simulink library "Renault_Library"  (Simulink 8.2)
settings: Solver=ode3  StartTime=0.0  StopTime=10.0  FixedStep=0.020  RelTol=1e-3
undefined symbols (not defined in the file or in its folder): ABC, AssystemProposal, b, chkval, CoeffA_C, CoeffB_C, CoeffMean_C, ConfTime_C, DiagDelay_C, DividePercent, FailureConfActive_C, FallingEdgeDuration_C, HighLevelThresholdVoltage_C, HighLevelVoltage_C, HighLevelVoltageTunable_C, InitValue_C, InputVoltRangeMax_C, InputVoltRangeMin_C, LowActiveContact_C, LowLevelThresholdVoltage_C, LowLevelVoltage_C, MaxOperatingVoltage_C, MinOperatingVoltage_C, mV2V_conversion, OUT1, OutOfRangeHigh_C, OutOfRangeLow_C, PinSupplySaturationMax, PinSupplySaturationMin, Res1Value_C, Res2Value_C, Res3Value_C, RisingEdgeDuration_C, RSA_CANSignalFailMonitoring, SampleTime_C, service14Table, service19Table, service22Table, service24Table, service28Table, service2ETable, service2FTable, service31Table, service87Table, SizeXStateDID2FTable, SizeXTable14, SizeXTable19, SizeXTable22, SizeXTable24, SizeXTable28, SizeXTable2E, SizeXTable2F, SizeXTable31, SizeXTable87, SizeYStateDID2FTable, SizeYTable14, SizeYTable19, SizeYTable22, SizeYTable24, SizeYTable28 ... (+22)
undefined symbols (not defined in the file): BatteryVoltage_ActivationThreshold_C, FrameStatus_AbsentValue_C, FrameStatus_ConfAbsentValue_C, MemThreshold_C, NAMEOFTHEMODEL_SAMPLE_TIME_CST, NbAbs1_C, NbConfAbs1_C, NbConfPres1_C, NbPres1_C, NOMDUSIGNAL_FaultConfTime_C, NOMDUSIGNAL_FaultRehabTime_C, NOMDUSIGNAL_switchvalue_C, NOMDUSIGNAL_unavailablevalue_C, PeriodFrame1_C, ResetTime_C, WakeUpTime_C
libraries: Renault_Library x112, simulink x75
(part 12 of 32)

== system Renault_Library/Complex blocks/Timers & Counters/RSA_TurnOnDelay2_v01_05 ==
  in : Inport
      settings: AttributesFormatString=Type : %<OutDataTypeStr>
  dly : Inport  Port=2
      settings: AttributesFormatString=Type : %<OutDataTypeStr>
  Constant1 : Constant  Value=Ts  OutDataTypeStr=Inherit: Inherit via back propagation  SampleTime=-1
  Constant4 : Constant  Tag=AM,Constant,0,1,Value;CMP,Value;  Value=1000  OutDataTypeStr=uint16  SampleTime=-1
  Constant5 : Constant  Value=INIVAL  OutDataTypeStr=Inherit: Inherit via back propagation  SampleTime=-1
  Constant6 : Constant  Tag=AM,Constant,0,1,Value;CMP,Value;  OutDataTypeStr=Inherit: Inherit via back propagation  SampleTime=-1
  DataTypeConversion1 : DataTypeConversion  OutDataTypeStr=uint32  SaturateOnIntegerOverflow=on  RndMeth=Zero
  DataTypeConversion2 : DataTypeConversion  RndMeth=Zero
  DataTypeConversion3 : DataTypeConversion  RndMeth=Zero
  Logical_Operator : Logic  Operator=NOT  OutDataTypeStr=boolean
  Logical_Operator1 : Logic  OutDataTypeStr=boolean
  Logical_Operator2 : Logic  OutDataTypeStr=boolean
  Logical_Operator6 : Logic  Operator=NOT  OutDataTypeStr=boolean
  Product : Product  SaturateOnIntegerOverflow=on  RndMeth=Zero
  Product1 : Product  SaturateOnIntegerOverflow=on  RndMeth=Zero
  Product2 : Product  Inputs=*/  SaturateOnIntegerOverflow=on  RndMeth=Zero
  RSA_CompareToZero : Renault_Library/Complex blocks/Logic & Bit Operations/RSA_CompareToZero  Tag=[LIB]  relop=~=  LogicOutDataTypeMode=boolean
      settings: AttributesFormatString=%<MaskType> : Ver : 01.03\nOperator: %<relop>\nOutput data type mode: %<LogicOutDataTypeMode>\nEnable zero-crossing detection %<ZeroCross>
  RSA_DataTypeDuplicate_v01_03 : Renault_Library/Complex blocks/Signal Routing/RSA_DataTypeDuplicate_v01_03  Tag=[LIB]  NoIn=2
      settings: AttributesFormatString=%<MaskType> : Ver : 01.03\nNo Of Inputs %<NoIn>
  Relational_Operator1 : RelationalOperator  Operator=<=  OutDataTypeStr=boolean  InputSameDT=on
  Relational_Operator8 : RelationalOperator  Operator=>  OutDataTypeStr=boolean  InputSameDT=on
  Subtract4 : Sum  Inputs=-+  OutDataTypeStr=Inherit: Inherit via back propagation  RndMeth=Zero  SaturateOnIntegerOverflow=on  InputSameDT=on
      settings: AttributesFormatString=SAT = %<SaturateOnIntegerOverflow>
  Sum1 : Sum  Inputs=+-  OutDataTypeStr=Inherit: Inherit via back propagation  RndMeth=Zero  SaturateOnIntegerOverflow=on  InputSameDT=on
      settings: AttributesFormatString=SAT = %<SaturateOnIntegerOverflow>
  Switch1 : Switch  Criteria=u2 ~= 0  RndMeth=Zero  SaturateOnIntegerOverflow=on  InputSameDT=on
      settings: AttributesFormatString=Criteria=%<Criteria>
  Switch2 : Switch  Criteria=u2 ~= 0  RndMeth=Zero  SaturateOnIntegerOverflow=on  InputSameDT=on
      settings: AttributesFormatString=Criteria=%<Criteria>
  Switch3 : Switch  Criteria=u2 ~= 0  RndMeth=Zero  SaturateOnIntegerOverflow=on  InputSameDT=on
      settings: AttributesFormatString=Criteria=%<Criteria>
  Switch5 : Switch  Tag=GEN,Threshold;AM,Switch,2,1;CMP,Threshold;  Criteria=u2 ~= 0  RndMeth=Zero  SaturateOnIntegerOverflow=on  InputSameDT=on
      settings: AttributesFormatString=Criteria=%<Criteria>
  UnitDelay : UnitDelay  InitialCondition=false  SampleTime=-1
      settings: AttributesFormatString=IC = %<X0>
  Unit_Delay2 : UnitDelay  InitialCondition=INIVAL  SampleTime=-1
      settings: AttributesFormatString=IC = %<X0>
  out : Outport
      settings: AttributesFormatString=Type : %<OutDataTypeStr>\nInit :%<InitialOutput>
  ctr : Outport  Port=2
      settings: AttributesFormatString=Type : %<OutDataTypeStr>\nInit :%<InitialOutput>
  wires:
  Constant5/1 -> [INIVAL], Switch1/1
  in/1 -> Logical_Operator1/1, UnitDelay/1, Logical_Operator2/1, Logical_Operator/1
  [dly] -> Switch2/1
  Logical_Operator2/1 -> Switch2/2
  Logical_Operator6/1 -> Logical_Operator2/2
  UnitDelay/1 -> Logical_Operator6/1
  Switch1/1 -> Product2/1, Unit_Delay2/1, Relational_Operator8/2
  Switch2/1 -> Switch1/3
  Logical_Operator1/1 -> out/1
  Relational_Operator8/1 -> Logical_Operator1/2
  Logical_Operator/1 -> Switch1/2
  Unit_Delay2/1 -> Sum1/1, Relational_Operator1/1
  Relational_Operator1/1 -> Switch3/2
  Sum1/1 -> Switch3/3
  [Timestep] -> Sum1/2
  Switch3/1 -> Switch2/3
  [INIVAL] -> Switch3/1
  [Ts] -> DataTypeConversion1/1, Subtract4/2
  DataTypeConversion1/1 -> DataTypeConversion2/1
  DataTypeConversion2/1 -> Subtract4/1
  RSA_CompareToZero/1 -> Switch5/2
  Subtract4/1 -> RSA_CompareToZero/1
  Constant6/1 -> Switch5/3
  Constant4/1 -> Switch5/1
  Switch5/1 -> [tolcons]
  Product/1 -> [Timestep]
  Constant1/1 -> RSA_DataTypeDuplicate_v01_03/2, [Ts], Product/1
  [tolcons] -> Product/2
  [Timestep] -> Relational_Operator1/2
  [Timestep] -> Relational_Operator8/1
  Product1/1 -> [dly]
  [tolcons] -> Product1/1
  dly/1 -> RSA_DataTypeDuplicate_v01_03/1, Product1/2
  DataTypeConversion3/1 -> ctr/1
  Product2/1 -> DataTypeConversion3/1
  [tolcons] -> Product2/2

== system Renault_Library/Complex blocks/Timers & Counters/RSA_TurnOnDelay2_v01_06 ==
  delay_flag : Inport
      settings: AttributesFormatString=Type : %<OutDataTypeStr>
  dly : Inport  Port=2
      settings: AttributesFormatString=Type : %<OutDataTypeStr>
  Constant1 : Constant  Value=Ts  OutDataTypeStr=SampleDT
  Constant2 : Constant  Value=Boosting_Factor  OutDataTypeStr=Constant_DT
  Constant5 : Constant  Value=INIVAL  OutDataTypeStr=Inherit: Inherit via back propagation
  Logical_Operator : Logic  Operator=NOT  OutDataTypeStr=boolean
  Logical_Operator1 : Logic  OutDataTypeStr=boolean
  Logical_Operator6 : Logic  Operator=NOT  OutDataTypeStr=boolean
  Product : Product  OutDataTypeStr=Inherit: Inherit via back propagation  SaturateOnIntegerOverflow=on  RndMeth=Zero
  Product1 : Product  OutDataTypeStr=Inherit: Inherit via back propagation  SaturateOnIntegerOverflow=on  RndMeth=Zero
  Product2 : Product  Inputs=*/  OutDataTypeStr=Inherit: Inherit via back propagation  SaturateOnIntegerOverflow=on  RndMeth=Zero
  Relational_Operator1 : RelationalOperator  Operator=<=  OutDataTypeStr=boolean  InputSameDT=on
  Relational_Operator8 : RelationalOperator  Operator=>  OutDataTypeStr=boolean  InputSameDT=on
  Sum1 : Sum  Inputs=+-  OutDataTypeStr=Inherit: Inherit via back propagation  RndMeth=Zero  SaturateOnIntegerOverflow=on  InputSameDT=on
      settings: AttributesFormatString=SAT = %<SaturateOnIntegerOverflow>
  Switch1 : Switch  Criteria=u2 ~= 0  RndMeth=Zero  SaturateOnIntegerOverflow=on  InputSameDT=on
      settings: AttributesFormatString=Criteria=%<Criteria>
  Switch2 : Switch  Criteria=u2 ~= 0  RndMeth=Zero  SaturateOnIntegerOverflow=on  InputSameDT=on
      settings: AttributesFormatString=Criteria=%<Criteria>
  Switch3 : Switch  Criteria=u2 ~= 0  RndMeth=Zero  SaturateOnIntegerOverflow=on  InputSameDT=on
      settings: AttributesFormatString=Criteria=%<Criteria>
  UnitDelay : UnitDelay  InitialCondition=false  SampleTime=-1
      settings: AttributesFormatString=IC = %<X0>
  Unit_Delay2 : UnitDelay  InitialCondition=INIVAL  SampleTime=-1
      settings: AttributesFormatString=IC = %<X0>
  delay_out : Outport
      settings: AttributesFormatString=Type : %<OutDataTypeStr>\nInit :%<InitialOutput>
  ctr : Outport  Port=2
      settings: AttributesFormatString=Type : %<OutDataTypeStr>\nInit :%<InitialOutput>
  wires:
  dly/1 -> Product1/1
  Constant5/1 -> [INIVAL], Switch1/1
  delay_flag/1 -> Logical_Operator1/1, UnitDelay/1, Logical_Operator/1
  [dly] -> Switch2/1
  Logical_Operator6/1 -> Switch2/2
  UnitDelay/1 -> Logical_Operator6/1
  Switch1/1 -> Relational_Operator8/2, Unit_Delay2/1, Product2/1
  Switch2/1 -> Switch1/3
  Logical_Operator1/1 -> delay_out/1
  Relational_Operator8/1 -> Logical_Operator1/2
  Logical_Operator/1 -> Switch1/2
  Unit_Delay2/1 -> Sum1/1, Relational_Operator1/1
  Relational_Operator1/1 -> Switch3/2
  Sum1/1 -> Switch3/3
  [Timestep] -> Sum1/2
  Switch3/1 -> Switch2/3
  [INIVAL] -> Switch3/1
  Constant1/1 -> Product/1
  [Timestep] -> Relational_Operator1/2
  [Timestep] -> Relational_Operator8/1
  Product2/1 -> ctr/1
  Product/1 -> [Timestep]
  Product1/1 -> [dly]
  [tolcons] -> Product2/2
  [tolcons] -> Product1/2
  [tolcons] -> Product/2
  Constant2/1 -> [tolcons]

== system Renault_Library/Complex blocks/Timers & Counters/RSA_TurnOnDelay_v01_03 ==
  Delaytime : Inport  Tag=AM,In,0,1,Name,C;CMP,Port,PortWidth,SampleTime;
      settings: AttributesFormatString=Type : %<OutDataTypeStr>
  Signal : Inport  Tag=AM,In,0,1,Name,C;CMP,Port,PortWidth,SampleTime;  Port=2
      settings: AttributesFormatString=Type : %<OutDataTypeStr>
  Timestep : Inport  Tag=AM,In,0,1,Name,C;CMP,Port,PortWidth,SampleTime;  Port=3
      settings: AttributesFormatString=Type : %<OutDataTypeStr>
  LogicalOperator : Logic  Tag=AM,Std,Inputs,1;CMP,Operator,Inputs;  OutDataTypeStr=boolean
  RSA_Timer1_v01_1 : Renault_Library/Complex blocks/Timers & Counters/RSA_Timer1_v01_04  Tag=[LIB]  timeoutinit=timeoutinit  initinit=initinit
      settings: AttributesFormatString=%<MaskType> : Ver : 01.04\nTimeout output initialisation=%<timeoutinit>\nInit input initialisation=%<initinit>
  Terminator : Terminator
  Out : Outport  Tag=AM,Out,1,0,Name,NC;CMP,Port,OutputWhenDisabled,InitialOutput;
      settings: AttributesFormatString=Type : %<OutDataTypeStr>\nInit :%<InitialOutput>
  wires:
  Delaytime/1 -> RSA_Timer1_v01_1/2
  RSA_Timer1_v01_1/2 -> Terminator/1  "Timer"
  Signal/1 -> RSA_Timer1_v01_1/1, LogicalOperator/1  "Signal"
  LogicalOperator/1 -> Out/1
  RSA_Timer1_v01_1/1 -> LogicalOperator/2  "Timeout"
  Timestep/1 -> RSA_Timer1_v01_1/3

== system Renault_Library/Complex blocks/Timers & Counters/RSA_TurnOnDelay_v01_04 ==
  Delaytime : Inport  Tag=AM,In,0,1,Name,C;CMP,Port,PortWidth,SampleTime;
      settings: AttributesFormatString=Type : %<OutDataTypeStr>
  Signal : Inport  Tag=AM,In,0,1,Name,C;CMP,Port,PortWidth,SampleTime;  Port=2
      settings: AttributesFormatString=Type : %<OutDataTypeStr>
  Timestep : Inport  Tag=AM,In,0,1,Name,C;CMP,Port,PortWidth,SampleTime;  Port=3
      settings: AttributesFormatString=Type : %<OutDataTypeStr>
  Constant1 : Constant  Tag=AM,Constant,0,1,Value;CMP,Value;  Value=TIM1INI  OutDataTypeStr=Inherit: Inherit via back propagation  SampleTime=-1
  Constant3 : Constant  Tag=AM,Constant,0,1,Value;CMP,Value;  Value=TIM1INI  OutDataTypeStr=Inherit: Inherit via back propagation  SampleTime=-1
  Constant4 : Constant  Tag=AM,Constant,0,1,Value;CMP,Value;  Value=1000  OutDataTypeStr=uint16  SampleTime=-1
  Constant5 : Constant  Tag=AM,Constant,0,1,Value;CMP,Value;  OutDataTypeStr=Inherit: Inherit via back propagation  SampleTime=-1
  DataTypeConversion1 : DataTypeConversion  OutDataTypeStr=uint32  SaturateOnIntegerOverflow=on  RndMeth=Zero
  DataTypeConversion2 : DataTypeConversion  RndMeth=Zero
  DelayInput1 : UnitDelay  Description=\nStore in Global RAM  InitialCondition=timeoutinit  SampleTime=-1
  DelayInput2 : UnitDelay  Description=\nStore in Global RAM  InitialCondition=TIM1INI  SampleTime=-1
  EdgeRising : Subsystem  mask(EdgeRising){vinit=initinit}
      mask prompts: vinit "Initialisation"
      mask help: When a rising edge is used as input, the ouput is equal to one during the fall of the signal. Otherwise the output is zero.
      mask Display:
      | plot([0.2 0.4 0.4 0.6],[0.6 0.6 0.9 0.9]);
      | plot([0.2 0.4 0.4 0.4 0.6],[0.1 0.1 0.4 0.1 0.1])
  LogicalOperator : Logic  Tag=AM,Std,Inputs,1;CMP,Operator,Inputs;  OutDataTypeStr=boolean
  LogicalOperator1 : Logic  OutDataTypeStr=boolean
  Product : Product  OutDataTypeStr=Inherit: Inherit via back propagation  SaturateOnIntegerOverflow=on  RndMeth=Zero
  Product1 : Product  OutDataTypeStr=Inherit: Inherit via back propagation  SaturateOnIntegerOverflow=on  RndMeth=Zero
  RSA_CompareToZero : Renault_Library/Complex blocks/Logic & Bit Operations/RSA_CompareToZero  Tag=[LIB]  SystemSampleTime=-1  relop=~=  LogicOutDataTypeMode=boolean
      settings: AttributesFormatString=%<MaskType> : Ver : 01.03\nOperator: %<relop>\nOutput data type mode: %<LogicOutDataTypeMode>\nEnable zero-crossing detection %<ZeroCross>
  RSA_DataTypeDuplicate_v01_03 : Renault_Library/Complex blocks/Signal Routing/RSA_DataTypeDuplicate_v01_03  Tag=[LIB]  SystemSampleTime=-1  NoIn=2
      settings: AttributesFormatString=%<MaskType> : Ver : 01.03\nNo Of Inputs %<NoIn>
  RelationalOperator1 : RelationalOperator  Operator=>  OutDataTypeStr=boolean  ZeroCross=off
  RelationalOperator2 : RelationalOperator  Operator=<=  OutDataTypeStr=boolean  ZeroCross=off  InputSameDT=on
  Subtract4 : Sum  Inputs=-+  OutDataTypeStr=Inherit: Inherit via back propagation  RndMeth=Zero  SaturateOnIntegerOverflow=on  InputSameDT=on
      settings: AttributesFormatString=SAT = %<SaturateOnIntegerOverflow>
  Sum : Sum  Inputs=+-  OutDataTypeStr=Inherit: Inherit via back propagation  RndMeth=Nearest  SaturateOnIntegerOverflow=on
      settings: AttributesFormatString=SAT = %<SaturateOnIntegerOverflow>
  Switch1 : Switch  Tag=GEN,Threshold;AM,Switch,2,1;CMP,Threshold;  Criteria=u2 ~= 0  RndMeth=Zero  SaturateOnIntegerOverflow=on  InputSameDT=on
      settings: AttributesFormatString=Criteria=%<Criteria>
  Switch2 : Switch  Tag=GEN,Threshold;AM,Switch,2,1;CMP,Threshold;  Criteria=u2 ~= 0  RndMeth=Zero  SaturateOnIntegerOverflow=on  InputSameDT=on
      settings: AttributesFormatString=Criteria=%<Criteria>
  Switch3 : Switch  Tag=GEN,Threshold;AM,Switch,2,1;CMP,Threshold;  Criteria=u2 ~= 0  RndMeth=Zero  SaturateOnIntegerOverflow=on
      settings: AttributesFormatString=Criteria=%<Criteria>
  Switch4 : Switch  Tag=GEN,Threshold;AM,Switch,2,1;CMP,Threshold;  Criteria=u2 ~= 0  OutDataTypeStr=Inherit: Inherit via back propagation  RndMeth=Zero  SaturateOnIntegerOverflow=on
      settings: AttributesFormatString=Criteria=%<Criteria>
  Switch5 : Switch  Tag=GEN,Threshold;AM,Switch,2,1;CMP,Threshold;  Criteria=u2 ~= 0  RndMeth=Zero  SaturateOnIntegerOverflow=on  InputSameDT=on
      settings: AttributesFormatString=Criteria=%<Criteria>
  Out : Outport  Tag=AM,Out,1,0,Name,NC;CMP,Port,OutputWhenDisabled,InitialOutput;
      settings: AttributesFormatString=Type : %<OutDataTypeStr>\nInit :%<InitialOutput>
  wires:
  [Timestep1] -> RelationalOperator2/1, Sum/2
  [EdgeRising] -> Switch2/2
  EdgeRising/1(Out) -> Switch1/2, Switch1/1, [EdgeRising]
  Switch1/1 -> DelayInput1/1, LogicalOperator1/1
  DelayInput1/1 -> Switch1/3
  RelationalOperator1/1 -> LogicalOperator1/2, Switch3/2
  Switch3/1 -> DelayInput2/1
  Switch2/1 -> Switch3/3, RelationalOperator1/2
  Switch4/1 -> Switch2/3
  RelationalOperator2/1 -> Switch4/2
  DelayInput2/1 -> Sum/1, RelationalOperator2/2
  Constant3/1 -> Switch3/1
  Constant1/1 -> Switch4/3
  Signal/1 -> LogicalOperator/1, EdgeRising/1(In)  "Signal"
  LogicalOperator/1 -> Out/1
  LogicalOperator1/1 -> LogicalOperator/2  "Timeout"
  Product/1 -> [Timestep1]
  Timestep/1 -> RSA_DataTypeDuplicate_v01_03/1, [Timestep], Product/1
  [tolcons] -> Product/2
  Delaytime/1 -> RSA_DataTypeDuplicate_v01_03/2, Product1/1
  [tolcons] -> Product1/2
  Product1/1 -> Switch2/1
  [Timestep1] -> RelationalOperator1/1
  Sum/1 -> Switch4/1
  [Timestep] -> DataTypeConversion1/1, Subtract4/2
  DataTypeConversion1/1 -> DataTypeConversion2/1
  DataTypeConversion2/1 -> Subtract4/1
  RSA_CompareToZero/1 -> Switch5/2
  Subtract4/1 -> RSA_CompareToZero/1
  Constant5/1 -> Switch5/3
  Constant4/1 -> Switch5/1
  Switch5/1 -> [tolcons]

== system Renault_Library/Complex blocks/Timers & Counters/RSA_TurnOnDelay_v01_05 ==
  Delaytime : Inport  Tag=AM,In,0,1,Name,C;CMP,Port,PortWidth,SampleTime;
      settings: AttributesFormatString=Type : %<OutDataTypeStr>
  Signal : Inport  Tag=AM,In,0,1,Name,C;CMP,Port,PortWidth,SampleTime;  Port=2
      settings: AttributesFormatString=Type : %<OutDataTypeStr>
  Timestep : Inport  Tag=AM,In,0,1,Name,C;CMP,Port,PortWidth,SampleTime;  Port=3
      settings: AttributesFormatString=Type : %<OutDataTypeStr>
  Constant1 : Constant  Tag=AM,Constant,0,1,Value;CMP,Value;  Value=TIM1INI  OutDataTypeStr=Inherit: Inherit via back propagation  SampleTime=-1
  Constant2 : Constant  Value=CONS_ONE  OutDataTypeStr=Inherit: Inherit via back propagation
  Constant3 : Constant  Value=TIM1INI  OutDataTypeStr=Inherit: Inherit via back propagation  SampleTime=-1
  Constant6 : Constant  Value=CONS_ZERO  OutDataTypeStr=Inherit: Inherit via back propagation
  Constant7 : Constant  Value=BOOSTING_FLP  OutDataTypeStr=uint32
  Constant8 : Constant  Value=BOOSTING_FXP  OutDataTypeStr=Inherit: Inherit via back propagation
  DataTypeConversion2 : DataTypeConversion  RndMeth=Zero
  DelayInput1 : UnitDelay  Description=\nStore in Global RAM  InitialCondition=timeoutinit  SampleTime=-1
      settings: AttributesFormatString=IC = %<X0>
  DelayInput2 : UnitDelay  Description=\nStore in Global RAM  InitialCondition=TIM1INI  SampleTime=-1
      settings: AttributesFormatString=IC = %<X0>
  EdgeRising : Subsystem  mask(EdgeRising){vinit=initinit}
      mask prompts: vinit "Initialisation"
      mask help: When a rising edge is used as input, the ouput is equal to one during the fall of the signal. Otherwise the output is zero.
      mask Display:
      | plot([0.2 0.4 0.4 0.6],[0.6 0.6 0.9 0.9]);
      | plot([0.2 0.4 0.4 0.4 0.6],[0.1 0.1 0.4 0.1 0.1])
  LogicalOperator : Logic  Tag=AM,Std,Inputs,1;CMP,Operator,Inputs;  OutDataTypeStr=boolean
  LogicalOperator1 : Logic  OutDataTypeStr=boolean
  Mux : Mux  Inputs=2
  Product : Product  OutDataTypeStr=Inherit: Inherit via back propagation  SaturateOnIntegerOverflow=on  RndMeth=Zero
  Product1 : Product  OutDataTypeStr=Inherit: Inherit via back propagation  SaturateOnIntegerOverflow=on  RndMeth=Zero
  Product2 : Product  OutDataTypeStr=uint32  SaturateOnIntegerOverflow=on  RndMeth=Zero
  RelationalOperator1 : RelationalOperator  Operator=>  OutDataTypeStr=boolean  ZeroCross=off  InputSameDT=on
  RelationalOperator2 : RelationalOperator  Operator=<=  OutDataTypeStr=boolean  ZeroCross=off  InputSameDT=on
  RelationalOperator3 : RelationalOperator  Operator=>  OutDataTypeStr=boolean  ZeroCross=off  InputSameDT=on
  Subtract4 : Sum  Inputs=-+  OutDataTypeStr=Inherit: Inherit via back propagation  RndMeth=Zero  InputSameDT=on
      settings: AttributesFormatString=SAT = %<SaturateOnIntegerOverflow>
  Sum : Sum  Inputs=+-  OutDataTypeStr=Inherit: Inherit via back propagation  RndMeth=Zero  InputSameDT=on
      settings: AttributesFormatString=SAT = %<SaturateOnIntegerOverflow>
  Switch{1..5} (5 alike) : Switch  Tag=GEN,Threshold;AM,Switch,2,1;CMP,Threshold;  Criteria=u2 ~= 0  RndMeth=Zero  SaturateOnIntegerOverflow=on  InputSameDT=on
      settings: AttributesFormatString=Criteria=%<Criteria>
  Terminator : Terminator
  Out : Outport  Tag=AM,Out,1,0,Name,NC;CMP,Port,OutputWhenDisabled,InitialOutput;
      settings: AttributesFormatString=Type : %<OutDataTypeStr>\nInit :%<InitialOutput>
  wires:
  [Timestep] -> Subtract4/2, Product2/2
  Timestep/1 -> [Timestep], Product/1, Mux/1
  Mux/1 -> Terminator/1
  [Timestep1] -> RelationalOperator2/1, Sum/2
  [EdgeRising] -> Switch2/2
  EdgeRising/1(Out) -> Switch1/2, Switch1/1, [EdgeRising]
  Switch1/1 -> DelayInput1/1, LogicalOperator1/1
  DelayInput1/1 -> Switch1/3
  RelationalOperator1/1 -> LogicalOperator1/2, Switch3/2
  Switch3/1 -> DelayInput2/1
  Switch2/1 -> Switch3/3, RelationalOperator1/2
  Switch4/1 -> Switch2/3
  RelationalOperator2/1 -> Switch4/2
  DelayInput2/1 -> Sum/1, RelationalOperator2/2
  Constant3/1 -> Switch3/1
  Constant1/1 -> Switch4/3
  Signal/1 -> LogicalOperator/1, EdgeRising/1(In)  "Signal"
  LogicalOperator/1 -> Out/1
  LogicalOperator1/1 -> LogicalOperator/2  "Timeout"
  Product/1 -> [Timestep1]
  [tolcons] -> Product/2
  Delaytime/1 -> Product1/1, Mux/2
  [tolcons] -> Product1/2
  Product1/1 -> Switch2/1
  [Timestep1] -> RelationalOperator1/1
  Sum/1 -> Switch4/1
  Product2/1 -> DataTypeConversion2/1
  DataTypeConversion2/1 -> Subtract4/1
  RelationalOperator3/1 -> Switch5/2
  Subtract4/1 -> RelationalOperator3/1
  Constant8/1 -> Switch5/3
  Constant7/1 -> Switch5/1
  Switch5/1 -> [tolcons]
  Constant2/1 -> Product2/1
  Constant6/1 -> RelationalOperator3/2

== system Renault_Library/Services ECU/Commonly_Used_Blocks/RSA_ServECU_Activation ==
  SupplyVoltage : Inport
  DataTypeConversion : DataTypeConversion  RndMeth=Zero
  LogicalOperator1 : Logic  OutDataTypeStr=boolean
  OperatingVoltageMax1 : Constant  Value=MaxOperatingVoltage_C  OutDataTypeStr=Inherit: Inherit via back propagation  SampleTime=-1
  OperatingVoltageMin1 : Constant  Value=MinOperatingVoltage_C  OutDataTypeStr=Inherit: Inherit via back propagation  SampleTime=-1
  RelationalOperator3 : RelationalOperator  OutDataTypeStr=boolean  InputSameDT=on
  RelationalOperator4 : RelationalOperator  Operator=<=  OutDataTypeStr=boolean  InputSameDT=on
  Enable : Outport
  wires:
  LogicalOperator1/1 -> Enable/1
  RelationalOperator3/1 -> LogicalOperator1/1
  RelationalOperator4/1 -> LogicalOperator1/2
  OperatingVoltageMax1/1 -> RelationalOperator4/2
  OperatingVoltageMin1/1 -> RelationalOperator3/2
  SupplyVoltage/1 -> DataTypeConversion/1
  DataTypeConversion/1 -> RelationalOperator4/1, RelationalOperator3/1

== system Renault_Library/Services ECU/Commonly_Used_Blocks/RSA_ServECU_EnaOutContact ==
  note: Filtered Output
  note: in1 = High Level Voltage
  note: in0 = Low Level Voltage
  note: Output with Falling/Rising Slopes
      | In parameters
  SupplyVoltage : Inport
  LogicalState : Inport  Port=2
  Enable : EnablePort  StatesWhenEnabling=reset
  ActiveStateAdaptation : Subsystem
  Constant : Constant  Value=0  OutDataTypeStr=Inherit: Inherit via back propagation  SampleTime=-1
  Constant1 : Constant  Value=0  OutDataTypeStr=Inherit: Inherit via back propagation  SampleTime=-1
  DataTypeConversion1 : DataTypeConversion  RndMeth=Zero
  DataTypeConversion2 : DataTypeConversion  RndMeth=Zero
  DataTypeConversion3 : DataTypeConversion  RndMeth=Zero
  FallTime1 : Constant  Value=FallingEdgeDuration_C  OutDataTypeStr=Inherit: Inherit via back propagation  SampleTime=-1
  LogicalOperator1 : Logic  Operator=OR  OutDataTypeStr=boolean
  LogicalOperator3 : Logic  Operator=NOT  OutDataTypeStr=boolean
  LowLevelVoltage : Constant  Value=LowLevelVoltage_C  OutDataTypeStr=Inherit: Inherit via back propagation  SampleTime=-1
  LowLevelVoltage3 : Constant  Value=HighLevelVoltageTunable_C  OutDataTypeStr=Inherit: Inherit via back propagation  SampleTime=-1
  LowLevelVoltage4 : Constant  Value=HighLevelVoltage_C  OutDataTypeStr=Inherit: Inherit via back propagation  SampleTime=-1
  RSA_ServECU_SoftwareFiltering : Renault_Library/Services ECU/Commonly_Used_Blocks/RSA_ServECU_SoftwareFiltering
  RelationalOperator : RelationalOperator  Operator=~=  OutDataTypeStr=boolean  InputSameDT=on
  RelationalOperator1 : RelationalOperator  Operator=~=  OutDataTypeStr=boolean  InputSameDT=on
  RiseTime1 : Constant  Value=RisingEdgeDuration_C  OutDataTypeStr=Inherit: Inherit via back propagation  SampleTime=-1
  Switch : Switch  Criteria=u2 ~= 0  RndMeth=Zero  InputSameDT=on
  Switch1 : Switch  Criteria=u2 ~= 0  RndMeth=Zero  InputSameDT=on
      settings: AttributesFormatString=Threshold : %<Threshold>\nSaturation:%<SaturateOnIntegerOverflow>
  SwitchWithSlope : Subsystem
  SwitchWithoutSlope : Subsystem
  Terminator : Terminator
  ms2s : Gain  Gain=.001  RndMeth=Zero
  ms2s1 : Gain  Gain=.001  RndMeth=Zero
  PhysicalOutput_F : Outport  OutputWhenDisabled=reset  InitialOutput=0
  wires:
  DataTypeConversion3/1 -> [SupplyVoltage]
  DataTypeConversion1/1 -> PhysicalOutput_F/1
  SupplyVoltage/1 -> DataTypeConversion3/1
  SwitchWithSlope/2(Out2) -> Terminator/1
  Switch/1 -> DataTypeConversion1/1
  SwitchWithSlope/1(Out1) -> Switch/3
  [LowLevelVoltage] -> SwitchWithoutSlope/3(In0)
  [HighLevelVoltage] -> SwitchWithoutSlope/1(In1)
  SwitchWithoutSlope/1(Out1) -> Switch/1
  LowLevelVoltage/1 -> [LowLevelVoltage], SwitchWithSlope/4(LowLevelVoltage)
  LogicalState/1 -> ActiveStateAdaptation/1(LogicalState)
  LogicalOperator3/1 -> SwitchWithoutSlope/Enable, Switch/2
  LogicalOperator1/1 -> LogicalOperator3/1, SwitchWithSlope/Enable
  RiseTime1/1 -> ms2s/1
  FallTime1/1 -> ms2s1/1
  ms2s/1 -> [RisingEdgeDuration_C], SwitchWithSlope/2(RiseTime)
  ms2s1/1 -> [FallingEdgeDuration_C], SwitchWithSlope/3(FallTime)
  Switch1/1 -> [HighLevelVoltage], SwitchWithSlope/5(HighLevelVoltage)
  DataTypeConversion2/1 -> SwitchWithoutSlope/2(Switch_Cmd), SwitchWithSlope/1(Switch_Cmd)
  ActiveStateAdaptation/1(AdaptedLogicalState) -> RSA_ServECU_SoftwareFiltering/1
  RSA_ServECU_SoftwareFiltering/1 -> DataTypeConversion2/1
  LowLevelVoltage4/1 -> Switch1/1
  LowLevelVoltage3/1 -> Switch1/2
  [SupplyVoltage] -> Switch1/3
  [FallingEdgeDuration_C] -> RelationalOperator1/1
  [RisingEdgeDuration_C] -> RelationalOperator/1
  RelationalOperator1/1 -> LogicalOperator1/2
  RelationalOperator/1 -> LogicalOperator1/1
  Constant/1 -> RelationalOperator/2
  Constant1/1 -> RelationalOperator1/2

== system Renault_Library/Services ECU/Commonly_Used_Blocks/RSA_ServECU_EnaOutContactSat ==
  note: Output with Falling/Rising Slopes
      | In parameters
  note: in0 = Low Level Voltage
  note: in1 = High Level Voltage
  SupplyVoltage : Inport
  LogicalState : Inport  Port=2
  Enable : EnablePort  StatesWhenEnabling=reset
  ActiveStateAdaptation : Subsystem
  Constant : Constant  Value=0  OutDataTypeStr=Inherit: Inherit via back propagation  SampleTime=-1
  Constant1 : Constant  Value=0  OutDataTypeStr=Inherit: Inherit via back propagation  SampleTime=-1
  DataTypeConversion1 : DataTypeConversion  RndMeth=Zero
  DataTypeConversion2 : DataTypeConversion  RndMeth=Zero
  DataTypeConversion3 : DataTypeConversion  RndMeth=Zero
  Divide3 : Product  Inputs=**/  RndMeth=Zero  InputSameDT=on
  FallTime1 : Constant  Value=FallingEdgeDuration_C  OutDataTypeStr=Inherit: Inherit via back propagation  SampleTime=-1
  LogicalOperator1 : Logic  Operator=OR  OutDataTypeStr=boolean
  LogicalOperator3 : Logic  Operator=NOT  OutDataTypeStr=boolean
  LowLevelVoltage : Constant  Value=LowLevelVoltage_C  OutDataTypeStr=Inherit: Inherit via back propagation  SampleTime=-1
  RSA_DetectionSaturation : Renault_Library/Complex blocks/Discontinuities/RSA_DetectionSaturation  Tag=[LIB]
  RSA_ServECU_SoftwareFiltering : Renault_Library/Services ECU/Commonly_Used_Blocks/RSA_ServECU_SoftwareFiltering
  RelationalOperator : RelationalOperator  Operator=~=  OutDataTypeStr=boolean  InputSameDT=on
  RelationalOperator1 : RelationalOperator  Operator=~=  OutDataTypeStr=boolean  InputSameDT=on
  Res1Value : Constant  Value=Res1Value_C  OutDataTypeStr=Inherit: Inherit via back propagation  SampleTime=-1
  Res2Value : Constant  Value=Res2Value_C  OutDataTypeStr=Inherit: Inherit via back propagation  SampleTime=-1
  Res3Value : Constant  Value=Res3Value_C  OutDataTypeStr=Inherit: Inherit via back propagation  SampleTime=-1
  RiseTime1 : Constant  Value=RisingEdgeDuration_C  OutDataTypeStr=Inherit: Inherit via back propagation  SampleTime=-1
  Subtract1 : Sum  RndMeth=Zero  InputSameDT=on
  Subtract2 : Sum  RndMeth=Zero  InputSameDT=on
  Subtract3 : Sum  Inputs=+-  RndMeth=Zero  InputSameDT=on
  Switch : Switch  Criteria=u2 ~= 0  RndMeth=Zero  InputSameDT=on
  SwitchWithSlope : Subsystem
  SwitchWithoutSlope : Subsystem
  Terminator : Terminator
  Terminator1 : Terminator
  data_1DLookupTable : Lookup_n-D  NumberOfTableDimensions=1  BreakpointsForDimension1=[0 3000 5999.9 6000 12000 16000]  Table=[0 0 0 800 2000 2000]  RndMeth=Zero  InputSameDT=on
  ms2s : Gain  Gain=.001  RndMeth=Zero
  ms2s1 : Gain  Gain=.001  RndMeth=Zero
  PhysicalOutput_F : Outport  OutputWhenDisabled=reset  InitialOutput=0
  wires:
  RSA_DetectionSaturation/2 -> Terminator1/1
  DataTypeConversion3/1 -> [SupplyVoltage]
  DataTypeConversion1/1 -> PhysicalOutput_F/1
  Subtract3/1 -> RSA_DetectionSaturation/3
  ms2s1/1 -> [FallingEdgeDuration_C], SwitchWithSlope/3(FallTime)
  ms2s/1 -> [RisingEdgeDuration_C], SwitchWithSlope/2(RiseTime)
  FallTime1/1 -> ms2s1/1
  RiseTime1/1 -> ms2s/1
  LogicalOperator1/1 -> LogicalOperator3/1, SwitchWithSlope/Enable
  LogicalOperator3/1 -> Switch/2, SwitchWithoutSlope/Enable
  LogicalState/1 -> ActiveStateAdaptation/1(LogicalState)
  RSA_DetectionSaturation/1 -> [HighLevelVoltage], SwitchWithSlope/5(HighLevelVoltage)
  [SupplyVoltage] -> RSA_DetectionSaturation/1
  LowLevelVoltage/1 -> [LowLevelVoltage], SwitchWithSlope/4(LowLevelVoltage)
  Subtract1/1 -> Divide3/3
  Res1Value/1 -> Subtract1/2
  Res2Value/1 -> Subtract2/1
  Res3Value/1 -> Subtract2/2
  Subtract2/1 -> Divide3/2, Subtract1/1
  Divide3/1 -> RSA_DetectionSaturation/2
  [SupplyVoltage] -> Divide3/1
  SwitchWithoutSlope/1(Out1) -> Switch/1
  [HighLevelVoltage] -> SwitchWithoutSlope/1(In1)
  [LowLevelVoltage] -> SwitchWithoutSlope/3(In0)
  SwitchWithSlope/1(Out1) -> Switch/3
  Switch/1 -> DataTypeConversion1/1
  SwitchWithSlope/2(Out2) -> Terminator/1
  SupplyVoltage/1 -> DataTypeConversion3/1
  [SupplyVoltage] -> Subtract3/1, data_1DLookupTable/1
  data_1DLookupTable/1 -> Subtract3/2
  ActiveStateAdaptation/1(AdaptedLogicalState) -> RSA_ServECU_SoftwareFiltering/1
  DataTypeConversion2/1 -> SwitchWithoutSlope/2(Switch_Cmd), SwitchWithSlope/1(Switch_Cmd)
  RSA_ServECU_SoftwareFiltering/1 -> DataTypeConversion2/1
  [FallingEdgeDuration_C] -> RelationalOperator1/1
  [RisingEdgeDuration_C] -> RelationalOperator/1
  RelationalOperator1/1 -> LogicalOperator1/2
  RelationalOperator/1 -> LogicalOperator1/1
  Constant/1 -> RelationalOperator/2
  Constant1/1 -> RelationalOperator1/2

== system Renault_Library/Services ECU/Commonly_Used_Blocks/RSA_ServECU_EnabledDiagnostic ==
  LogicalState : Inport
  Outputfeedback : Inport  Port=2
  DataTypeConversion : DataTypeConversion  RndMeth=Zero
  DataTypeConversion2 : DataTypeConversion  RndMeth=Zero
  DiagDelaySubsystem : Subsystem
  Divide : Product  Inputs=**/  RndMeth=Zero  InputSameDT=on
  FailureConfActive : Constant  Value=FailureConfActive_C  OutDataTypeStr=boolean  SampleTime=-1
  RSA_ServECU_FaultConfirmation : Renault_Library/Services ECU/Commonly_Used_Blocks/RSA_ServECU_FaultConfirmation
  RelationalOperator{1..3,7} (4 alike) : RelationalOperator  Operator=<  OutDataTypeStr=boolean  InputSameDT=on
  Res2Value : Constant  Value=Res2Value_C  OutDataTypeStr=Inherit: Inherit via back propagation  SampleTime=-1
  Res3Value : Constant  Value=Res3Value_C  OutDataTypeStr=Inherit: Inherit via back propagation  SampleTime=-1
  State_InfVoffMin : Constant  Value=State_InfVoffMin_C  OutDataTypeStr=Inherit: Inherit via back propagation  SampleTime=-1
  State_InfVonMin : Constant  Value=State_InfVonMin_C  OutDataTypeStr=Inherit: Inherit via back propagation  SampleTime=-1
  State_SupVoffMax_C : Constant  Value=State_SupVoffMax_C  OutDataTypeStr=Inherit: Inherit via back propagation  SampleTime=-1
  State_SupVonMax : Constant  Value=State_SupVonMax_C  OutDataTypeStr=Inherit: Inherit via back propagation  SampleTime=-1
  State_Voff : Constant  Value=State_Voff_C  OutDataTypeStr=Inherit: Inherit via back propagation  SampleTime=-1
  State_Von : Constant  Value=State_Von_C  OutDataTypeStr=Inherit: Inherit via back propagation  SampleTime=-1
  Subtract : Sum  RndMeth=Zero  InputSameDT=on
  Switch{1..5} (5 alike) : Switch  Criteria=u2 ~= 0  RndMeth=Zero  InputSameDT=on
  UnitDelay : UnitDelay  InitialCondition=InitValue_C  SampleTime=-1
  Voff_max : Constant  Value=VoffMax_C  OutDataTypeStr=Inherit: Inherit via back propagation  SampleTime=-1
  Voff_min : Constant  Value=VoffMin_C  OutDataTypeStr=Inherit: Inherit via back propagation  SampleTime=-1
  Von_max : Constant  Value=VonMin_C  OutDataTypeStr=Inherit: Inherit via back propagation  SampleTime=-1
  Von_min : Constant  Value=VonMax_C  OutDataTypeStr=Inherit: Inherit via back propagation  SampleTime=-1
  InternalFeedback : Outport  OutputWhenDisabled=reset  InitialOutput=0
  Diagnostic : Outport  Port=2  OutputWhenDisabled=reset  InitialOutput=0
  wires:
  State_SupVonMax/1 -> Switch3/3
  Divide/1 -> InternalFeedback/1, RelationalOperator3/1, RelationalOperator7/1, RelationalOperator2/1, RelationalOperator1/1
  Outputfeedback/1 -> DataTypeConversion/1
  Res2Value/1 -> Subtract/2
  Subtract/1 -> Divide/3
  Res3Value/1 -> Subtract/1, Divide/2
  State_SupVoffMax_C/1 -> Switch5/3
  Switch5/1 -> Switch4/3
  State_Voff/1 -> Switch5/1
  RelationalOperator3/1 -> Switch5/2
  Voff_max/1 -> RelationalOperator3/2
  Switch4/1 -> Switch1/3
  State_InfVoffMin/1 -> Switch4/1
  RelationalOperator7/1 -> Switch4/2
  Voff_min/1 -> RelationalOperator7/2
  State_Von/1 -> Switch3/1
  Switch3/1 -> Switch2/3
  RelationalOperator2/1 -> Switch3/2
  State_InfVonMin/1 -> Switch2/1
  Switch2/1 -> Switch1/1
  RelationalOperator1/1 -> Switch2/2
  Von_min/1 -> RelationalOperator2/2
  Von_max/1 -> RelationalOperator1/2
  LogicalState/1 -> DiagDelaySubsystem/1(In1)
  DataTypeConversion/1 -> Divide/1
  Switch1/1 -> RSA_ServECU_FaultConfirmation/2
  RSA_ServECU_FaultConfirmation/1 -> Diagnostic/1, UnitDelay/1
  FailureConfActive/1 -> RSA_ServECU_FaultConfirmation/3
  UnitDelay/1 -> RSA_ServECU_FaultConfirmation/1
  DataTypeConversion2/1 -> Switch1/2
  DiagDelaySubsystem/1(Out1) -> DataTypeConversion2/1

== system Renault_Library/Services ECU/Commonly_Used_Blocks/RSA_ServECU_EnabledInputContact ==
  SwitchVoltage : Inport
  Enable : EnablePort  StatesWhenEnabling=reset
  DataTypeConversion : DataTypeConversion  RndMeth=Zero
  DataTypeConversion1 : DataTypeConversion  RndMeth=Zero
  DataTypeConversion2 : DataTypeConversion  RndMeth=Zero
  LogicalOperator : Logic  Operator=NOR  OutDataTypeStr=boolean
  RSA_ServECU_FaultConfirmation : Renault_Library/Services ECU/Commonly_Used_Blocks/RSA_ServECU_FaultConfirmation
  RSA_ServECU_SoftwareFiltering : Renault_Library/Services ECU/Commonly_Used_Blocks/RSA_ServECU_SoftwareFiltering
  RelationalOperator : RelationalOperator  Operator===  OutDataTypeStr=boolean  InputSameDT=on
  RelationalOperator1 : RelationalOperator  Operator===  OutDataTypeStr=boolean  InputSameDT=on
  RelationalOperator{3,5..7} (4 alike) : RelationalOperator  Operator=<  OutDataTypeStr=boolean  InputSameDT=on
  State_InfVonMin : Constant  Value=State_InfVonMin_C  OutDataTypeStr=Inherit: Inherit via back propagation  SampleTime=-1
  State_SupVoffMax_C : Constant  Value=State_SupVoffMax_C  OutDataTypeStr=Inherit: Inherit via back propagation  SampleTime=-1
  State_SupVonMaxInfVoffmin : Constant  Value=State_SupVonMaxInfVoffmin_C  OutDataTypeStr=Inherit: Inherit via back propagation  SampleTime=-1
  State_Voff : Constant  Value=State_Voff_C  OutDataTypeStr=Inherit: Inherit via back propagation  SampleTime=-1
  State_Von : Constant  Value=State_Von_C  OutDataTypeStr=Inherit: Inherit via back propagation  SampleTime=-1
  Switch{,2..5} (5 alike) : Switch  Criteria=u2 ~= 0  RndMeth=Zero  InputSameDT=on
  UnitDelay : UnitDelay  InitialCondition=InitValue_C  SampleTime=-1
  Voff_max : Constant  Value=VoffMax_C  OutDataTypeStr=Inherit: Inherit via back propagation  SampleTime=-1
  Voff_min : Constant  Value=VoffMin_C  OutDataTypeStr=Inherit: Inherit via back propagation  SampleTime=-1
  Von_max : Constant  Value=VonMax_C  OutDataTypeStr=Inherit: Inherit via back propagation  SampleTime=-1
  Von_min : Constant  Value=VonMin_C  OutDataTypeStr=Inherit: Inherit via back propagation  SampleTime=-1
  SwitchState : Outport  OutputWhenDisabled=reset  InitialOutput=0
  SwitchState_F : Outport  Port=2  OutputWhenDisabled=reset  InitialOutput=0
  wires:
  State_Voff/1 -> [State_Voff]
  State_Von/1 -> [State_Von]
  SwitchVoltage/1 -> DataTypeConversion2/1
  State_SupVoffMax_C/1 -> Switch5/3
  Switch5/1 -> Switch4/3
  [State_Voff] -> Switch5/1
  RelationalOperator3/1 -> Switch5/2
  Voff_max/1 -> RelationalOperator3/2
  Switch4/1 -> Switch3/3
  State_SupVonMaxInfVoffmin/1 -> Switch4/1
  RelationalOperator7/1 -> Switch4/2
  Voff_min/1 -> RelationalOperator7/2
  [State_Von] -> Switch3/1
  Switch3/1 -> Switch2/3
  RelationalOperator6/1 -> Switch3/2
  State_InfVonMin/1 -> Switch2/1
  RelationalOperator5/1 -> Switch2/2
  Von_min/1 -> RelationalOperator5/2
  Von_max/1 -> RelationalOperator6/2
  Switch2/1 -> RSA_ServECU_FaultConfirmation/2, DataTypeConversion/1, RelationalOperator1/1, RSA_ServECU_SoftwareFiltering/1, RelationalOperator/1
  DataTypeConversion/1 -> SwitchState/1
  DataTypeConversion1/1 -> SwitchState_F/1
  DataTypeConversion2/1 -> RelationalOperator3/1, RelationalOperator7/1, RelationalOperator6/1, RelationalOperator5/1
  [State_Von] -> RelationalOperator/2
  [State_Voff] -> RelationalOperator1/2
  RelationalOperator/1 -> LogicalOperator/1
  RelationalOperator1/1 -> LogicalOperator/2
  LogicalOperator/1 -> Switch/2, RSA_ServECU_FaultConfirmation/3
  Switch/1 -> UnitDelay/1, DataTypeConversion1/1
  RSA_ServECU_SoftwareFiltering/1 -> Switch/3
  RSA_ServECU_FaultConfirmation/1 -> Switch/1
  UnitDelay/1 -> RSA_ServECU_FaultConfirmation/1

== system Renault_Library/Services ECU/Commonly_Used_Blocks/RSA_ServECU_EnabledSupply ==
  InputVoltage : Inport
  Enable : EnablePort  StatesWhenEnabling=reset
  CoeffA : Constant  Value=CoeffA_C  OutDataTypeStr=Inherit: Inherit via back propagation
  CoeffB : Constant  Value=CoeffB_C  OutDataTypeStr=Inherit: Inherit via back propagation
  DataTypeConversion : DataTypeConversion  RndMeth=Zero
  DataTypeConversion1 : DataTypeConversion  RndMeth=Zero
  DataTypeConversion2 : DataTypeConversion  RndMeth=Zero
  InputVoltRangeMax : Constant  Value=InputVoltRangeMax_C  OutDataTypeStr=Inherit: Inherit via back propagation
  InputVoltRangeMin : Constant  Value=InputVoltRangeMin_C  OutDataTypeStr=Inherit: Inherit via back propagation
  LogicalOperator1 : Logic  Operator=OR  OutDataTypeStr=boolean
  Mean : Subsystem
  OutOfRangeHigh : Constant  Value=OutOfRangeHigh_C  OutDataTypeStr=Inherit: Inherit via back propagation
  OutOfRangeLow : Constant  Value=OutOfRangeLow_C  OutDataTypeStr=Inherit: Inherit via back propagation
  Product : Product  OutDataTypeStr=Inherit: Inherit via back propagation  RndMeth=Zero  InputSameDT=on
      settings: AttributesFormatString=Saturation:%<SaturateOnIntegerOverflow>
  RSA_ServECU_SoftwareFiltering : Renault_Library/Services ECU/Commonly_Used_Blocks/RSA_ServECU_SoftwareFiltering
  RelationalOperator : RelationalOperator  Operator=<=  OutDataTypeStr=boolean  InputSameDT=on
  RelationalOperator1 : RelationalOperator  OutDataTypeStr=boolean  InputSameDT=on
  Sum : Sum  OutDataTypeStr=Inherit: Inherit via back propagation  RndMeth=Zero  InputSameDT=on
      settings: AttributesFormatString=Saturation: %<SaturateOnIntegerOverflow>
  Switch1 : Switch  Criteria=u2 ~= 0  RndMeth=Zero  InputSameDT=on
  Switch2 : Switch  Criteria=u2 ~= 0  RndMeth=Zero  InputSameDT=on
  OutputVoltage : Outport  OutputWhenDisabled=reset  InitialOutput=0
  OutputVoltage_F : Outport  Port=2  OutputWhenDisabled=reset  InitialOutput=0
  MeanOutputVoltage : Outport  Port=3  OutputWhenDisabled=reset  InitialOutput=0
  wires:
  Switch2/1 -> DataTypeConversion/1, RSA_ServECU_SoftwareFiltering/1, Mean/1(Output)
  Switch1/1 -> Switch2/1
  OutOfRangeHigh/1 -> Switch1/3
  RelationalOperator1/1 -> LogicalOperator1/2
  LogicalOperator1/1 -> Switch2/2
  Sum/1 -> Switch2/3
  RSA_ServECU_SoftwareFiltering/1 -> DataTypeConversion1/1
  DataTypeConversion/1 -> OutputVoltage/1
  DataTypeConversion1/1 -> OutputVoltage_F/1
  CoeffA/1 -> Product/2
  CoeffB/1 -> Sum/2
  Product/1 -> Sum/1
  InputVoltage/1 -> Product/1, RelationalOperator1/1, RelationalOperator/1
  RelationalOperator/1 -> Switch1/2, LogicalOperator1/1
  OutOfRangeLow/1 -> Switch1/1
  InputVoltRangeMin/1 -> RelationalOperator/2
  InputVoltRangeMax/1 -> RelationalOperator1/2
  Mean/1(MeanOutputVoltage) -> DataTypeConversion2/1
  DataTypeConversion2/1 -> MeanOutputVoltage/1

== system Renault_Library/Services ECU/Commonly_Used_Blocks/RSA_ServECU_EnabledSwitch ==
  SwitchVoltage : Inport
  Enable : EnablePort  StatesWhenEnabling=reset
  DataTypeConversion : DataTypeConversion  RndMeth=Zero
  DataTypeConversion1 : DataTypeConversion  RndMeth=Zero
  DataTypeConversion2 : DataTypeConversion  RndMeth=Zero
  RSA_ServECU_SoftwareFiltering : Renault_Library/Services ECU/Commonly_Used_Blocks/RSA_ServECU_SoftwareFiltering
  Relay : Relay  OnSwitchValue=HighLevelThresholdVoltage_C  OffSwitchValue=LowLevelThresholdVoltage_C  OnOutputValue=HighLevelOutput_C  OffOutputValue=LowLevelOutput_C
      settings: AttributesFormatString=<low=(%<offswitchvalue>,%<offoutputvalue>)>\n<high=(%<onswitchvalue>,%<onoutputvalue>)>\n
  LogicalState : Outport  OutputWhenDisabled=reset  InitialOutput=0
  LogicalState_F : Outport  Port=2  OutputWhenDisabled=reset  InitialOutput=0
  wires:
  RSA_ServECU_SoftwareFiltering/1 -> DataTypeConversion1/1
  SwitchVoltage/1 -> DataTypeConversion2/1
  Relay/1 -> DataTypeConversion/1, RSA_ServECU_SoftwareFiltering/1
  DataTypeConversion/1 -> LogicalState/1
  DataTypeConversion1/1 -> LogicalState_F/1
  DataTypeConversion2/1 -> Relay/1

== system Renault_Library/Services ECU/Commonly_Used_Blocks/RSA_ServECU_FaultConfirmation ==
  LastConfirmedState : Inport
  CurrentFaultState : Inport  Port=2
  Failure : Inport  Port=3
  FailureMonitoring : Subsystem
      settings: CopyFcn=set_param(gcb,'LinkStatus','inactive')
  Switch6 : Switch  Criteria=u2 ~= 0  RndMeth=Zero  InputSameDT=on
  ConfirmedFaultState : Outport
  wires:
  LastConfirmedState/1 -> Switch6/3
  Switch6/1 -> ConfirmedFaultState/1
  Failure/1 -> FailureMonitoring/2(Failure)  "Failure"
  CurrentFaultState/1 -> FailureMonitoring/1(CurrentFaultState)  "CurrentFaultState"
  FailureMonitoring/2(failure_flag) -> Switch6/2
  FailureMonitoring/1(ConfirmedFaultValue) -> Switch6/1  "ConfirmedFaultValue"

== system Renault_Library/Services ECU/Commonly_Used_Blocks/RSA_ServECU_SmartFET ==
  PwmRatio : Inport
  PinSupply : Inport  Port=2
  NoSmartProtection : Inport  Port=3
  Enable : EnablePort
  DataTypeConversion2 : DataTypeConversion  RndMeth=Zero
  DataTypeConversion3 : DataTypeConversion  OutDataTypeStr=uint8  RndMeth=Zero
  Divide2 : Product  Inputs=*/*  OutDataTypeStr=double  SaturateOnIntegerOverflow=on  RndMeth=Zero
  Divide3 : Product  Inputs=*/  OutDataTypeStr=double  SaturateOnIntegerOverflow=on  RndMeth=Zero
  DividePercent : Constant  Value=DividePercent  OutDataTypeStr=double
  DividePercent1 : Constant  Value=DividePercent  OutDataTypeStr=double
  Gain : Gain  Gain=mV2V_conversion  RndMeth=Zero
  Gain1 : Gain  Gain=mV2V_conversion  RndMeth=Zero
  Product1 : Product  OutDataTypeStr=double  RndMeth=Zero
  Saturation1 : Saturate  UpperLimit=PinSupplySaturationMax  LowerLimit=PinSupplySaturationMin  RndMeth=Zero
  SmartProtectionOn_Zero : Constant  Value=SmartProtectionOn_Zero  OutDataTypeStr=double
  Switch1 : Switch  Criteria=u2 ~= 0  OutDataTypeStr=double  RndMeth=Zero  InputSameDT=on
  PhysicalOutput : Outport  OutputWhenDisabled=reset  InitialOutput=0
  OutputCmd : Outport  Port=2  OutputWhenDisabled=reset  InitialOutput=0
  wires:
  DividePercent1/1 -> Divide2/3
  DataTypeConversion2/1 -> Divide3/1
  Divide3/1 -> Product1/2
  Divide2/1 -> DataTypeConversion3/1
  SmartProtectionOn_Zero/1 -> Switch1/3
  Product1/1 -> Switch1/1
  DataTypeConversion3/1 -> OutputCmd/1
  Switch1/1 -> PhysicalOutput/1, Gain1/1
  Saturation1/1 -> Divide2/2
  NoSmartProtection/1 -> Switch1/2
  PwmRatio/1 -> DataTypeConversion2/1
  PinSupply/1 -> [PinSupply], Product1/1
  DividePercent/1 -> Divide3/2
  [PinSupply] -> Gain/1
  Gain/1 -> Saturation1/1
  Gain1/1 -> Divide2/1

== system Renault_Library/Services ECU/Commonly_Used_Blocks/RSA_ServECU_SoftwareFiltering ==
  raw_value : Inport
  DataTypeConversion2 : DataTypeConversion  RndMeth=Zero
  Gain : Gain  Gain=0.001  RndMeth=Zero
      settings: AttributesFormatString=Saturation:%<SaturateOnIntegerOverflow>
  Gain1 : Gain  Gain=0.001  RndMeth=Zero
      settings: AttributesFormatString=Saturation:%<SaturateOnIntegerOverflow>
  InitValue : Constant  Value=InitValue_C  OutDataTypeStr=Inherit: Inherit via back propagation  SampleTime=-1
  SWFilteringChart : Stateflow chart  TreatAsAtomicUnit=on
  SampleTime : Constant  Value=SampleTime_C  OutDataTypeStr=Inherit: Inherit via back propagation  SampleTime=-1
  Tstab : Constant  Value=Tstab_C  OutDataTypeStr=Inherit: Inherit via back propagation  SampleTime=-1
  filtered_value : Outport
      settings: AttributesFormatString=Init :%<InitialOutput>
  wires:
  DataTypeConversion2/1 -> SWFilteringChart/1(raw_value)
  Tstab/1 -> Gain/1
  SWFilteringChart/1(filtered_value) -> filtered_value/1
  raw_value/1 -> DataTypeConversion2/1
  SampleTime/1 -> Gain1/1
  Gain/1 -> SWFilteringChart/2(Filter)
  Gain1/1 -> SWFilteringChart/3(SampleTime)
  InitValue/1 -> SWFilteringChart/4(InitValue)

== system Renault_Library/Services ECU/Diag/RSA_DiagnosticManagement ==
  DTOOL_TO_ECU_RECEIVED : Inport  OutDataTypeStr=uint8
  PART1_DTOOL_TO_ECU : Inport  Port=2  OutDataTypeStr=uint32
  PART2_DTOOL_TO_ECU : Inport  Port=3  OutDataTypeStr=uint32
  DIAG_INPUT : Inport  Port=4  OutDataTypeStr=Bus: busInput
  DIAGNOSTIC_ACTION_TOP : Subsystem
  DIAGNOSTIC_SANCTION_TOP : Subsystem
  DIAGNOSTIC_SEGMENTATION_TOP : Subsystem
  ECU_TO_DTOOL_RECEIVED : Outport  OutDataTypeStr=uint8
  PART1_ECU_TO_DTOOL : Outport  Port=2  OutDataTypeStr=uint32
  PART2_ECU_TO_DTOOL : Outport  Port=3  OutDataTypeStr=uint32
  DIAG_OUTPUT : Outport  Port=4  OutDataTypeStr=Bus: busOutput
  wires:
  DIAGNOSTIC_SEGMENTATION_TOP/2(PART1_ECU_TO_DTOOL) -> PART1_ECU_TO_DTOOL/1
  DIAGNOSTIC_SEGMENTATION_TOP/3(PART2_ECU_TO_DTOOL) -> PART2_ECU_TO_DTOOL/1
  DIAGNOSTIC_SEGMENTATION_TOP/1(ECU_TO_DTOOL_SEND) -> ECU_TO_DTOOL_RECEIVED/1
  [DIAG_BUFFER_INPUT] -> DIAGNOSTIC_SEGMENTATION_TOP/4(DIAG_BUFFER_RESPONSE)
  DIAGNOSTIC_ACTION_TOP/2(DIAG_BUFFER_RESPONSE) -> [DIAG_BUFFER_INPUT]
  DTOOL_TO_ECU_RECEIVED/1 -> DIAGNOSTIC_SEGMENTATION_TOP/1(DTOOL_TO_ECU_FRAME_RECEIVED)
  PART1_DTOOL_TO_ECU/1 -> DIAGNOSTIC_SEGMENTATION_TOP/2(PART1_DTOOL_TO_ECU)
  PART2_DTOOL_TO_ECU/1 -> DIAGNOSTIC_SEGMENTATION_TOP/3(PART2_DTOOL_TO_ECU)
  DIAG_INPUT/1 -> DIAGNOSTIC_ACTION_TOP/6(DIAG_INPUT)
  DIAGNOSTIC_ACTION_TOP/3(RES_DATA_NUMBER) -> [RES_DATA_NUMBER]
  [RES_DATA_NUMBER] -> DIAGNOSTIC_SEGMENTATION_TOP/5(RES_DATA_NUMBER)
  DIAGNOSTIC_SEGMENTATION_TOP/5(REQ_DATA_NUMBER) -> DIAGNOSTIC_SANCTION_TOP/2(REQ_DATA_NUMBER)
  DIAGNOSTIC_SEGMENTATION_TOP/6(SEG_STATE) -> DIAGNOSTIC_SANCTION_TOP/3(SEG_STATE)
  DIAGNOSTIC_SANCTION_TOP/1(NEGATIVE_RES_CODE) -> DIAGNOSTIC_ACTION_TOP/2(NEGATIVE_RES_CODE)
  DIAGNOSTIC_SANCTION_TOP/2(SUPPRESS_POS_RES) -> DIAGNOSTIC_ACTION_TOP/3(SUPPRESS_POS_RES)
  DIAGNOSTIC_SANCTION_TOP/3(SEND_RES_MESSAGE) -> DIAGNOSTIC_ACTION_TOP/4(SEND_RES_MESSAGE)
  DIAGNOSTIC_SANCTION_TOP/4(LIST_DID_SERVICE_22) -> DIAGNOSTIC_ACTION_TOP/5(LIST_DID_SERVICE_22)
  DIAGNOSTIC_SEGMENTATION_TOP/4(DIAG_BUFFER_REQUEST) -> DIAGNOSTIC_ACTION_TOP/1(DIAG_BUFFER_REQUEST), DIAGNOSTIC_SANCTION_TOP/1(DIAG_BUFFER_REQUEST)
  DIAGNOSTIC_ACTION_TOP/1(DIAG_OUTPUT) -> DIAG_OUTPUT/1
